simulink model "wlan_phy_rx_pmd"  (Simulink 7.8)
settings: Solver=VariableStepDiscrete  SolverMode=SingleTasking  StartTime=0.0  StopTime=rx_sim.sim_time  RelTol=1e-3
PostLoadFcn:
  | wlan_phy_rx_init
InitFcn:
  | wlan_phy_rx_init
StartFcn:
  | wlan_phy_rx_init
values from neighbouring files (symbols this model uses):
  barker_seq20 = 0.95 * circshift(barker_seq20, [0 4]) ./ max(abs(barker_seq20))    [wlan_phy_rx_init.m]
  bit_scrambler_lfsr_bytes = sum(bit_scrambler_lfsr_bytes .* repmat(2.^[0:7], 127, 1),2)    [wlan_phy_rx_init.m]
  longCorr_coef_i = [3*real(longCorr_coef)]    [wlan_phy_rx_init.m]
  longCorr_coef_q = [3*imag(longCorr_coef)]    [wlan_phy_rx_init.m]
  MAX_CP_LEN = 32    [wlan_phy_rx_init.m]
  MAX_NCBPS = NCBPS_11n_64QAM    (= 312)    [wlan_phy_rx_init.m]
  MAX_NUM_BYTES = 2^16-1    (= 65535)    [wlan_phy_rx_init.m]
  MAX_NUM_SC = 64    [wlan_phy_rx_init.m]
  MAX_NUM_SYMS = 600    [wlan_phy_rx_init.m]
  NDBPS_11a_BPSK12 = 24    [wlan_phy_rx_init.m]
  preFFT_sampBuff_numSamps = 4*MAX_NUM_SC    (= 256)    [wlan_phy_rx_init.m]
  rx_sim = struct()    [wlan_phy_rx_init.m]
  scr_ind_rev = zeros(1,128)    [wlan_phy_rx_init.m]
undefined symbols (not defined in the file or in its folder): gcbh, mcs_rom_11ag, mcs_rom_11n, wlan_rx_deinterleave_rom_gen, xlBlockLoadCallback, xlGetNormalizedPeriod, xlipgetpartsetting
libraries: xbsIndex_r4 x4943, xbsEDKLib_r4 x1
(part 18 of 28)

== system wlan_phy_rx_pmd/Sync & Antenna Sel/Pkt Det/RF B Det/Auto-Corr Pkt Det ==
  Reset OFDM : Inport
  Reset DSSS : Inport  Port=2
  rx_I : Inport  Port=3
  rx_Q : Inport  Port=4
  rx_valid : Inport  Port=5
  Auto Corr : Subsystem
  Auto Corr Pkt Det : Scope  NumInputPorts=9
      settings: TimeRange=40000  YMin=-1~-0.5~0~0~0~0~-1~0~0  YMax=1~0.5~1.5~1~0.4~1~1~1~1  SaveName=ScopeData28
  Decision : Subsystem
  Mag : Subsystem
  Register1 : xbsIndex_r4/Register  init=0  rst=off  en=off  dbl_ovrd=off  block_type=register
  Register2 : xbsIndex_r4/Register  init=0  rst=off  en=off  dbl_ovrd=off  block_type=register
  Register3 : xbsIndex_r4/Register  init=0  rst=off  en=off  dbl_ovrd=off  block_type=register
  done{1..9} (9 alike) : xbsIndex_r4/Gateway Out  inherit_from_input=off  hdl_port=off  timing_constraint=None  locs_specified=off  LOCs={}  UseAsDAC=off  DACChannel='1'  block_type=gatewayout
      settings: infoedit="Gateway out block.  Converts Xilinx fixed point inputs into ouputs of type Simulink integer, double, or fixed point.<P><P>Hardware notes:  In hardware these blocks become top level output ports or are discarded, depending on how they are configured."  xl_area=[0 0 0 0 1 0 0]  block_version=VER_STRING_GOES_HERE
  Det OFDM : Outport
  Det DSSS : Outport  Port=2
  wires:
  Reset DSSS/1 -> done9/1, Decision/2(Reset DSSS)
  Reset OFDM/1 -> done1/1, Decision/1(Reset OFDM)
  Decision/2(Det DSSS) -> Det DSSS/1, done8/1
  done9/1 -> Auto Corr Pkt Det/9  "Det Reset DSSS"
  done1/1 -> Auto Corr Pkt Det/8  "Det Reset OFDM"
  done8/1 -> Auto Corr Pkt Det/7  "Det DSSS"
  done6/1 -> Auto Corr Pkt Det/6  "Det OFDM"
  done7/1 -> Auto Corr Pkt Det/5  "Corr DSSS"
  done5/1 -> Auto Corr Pkt Det/4  "Corr OFDM"
  done3/1 -> Auto Corr Pkt Det/3  "Mag"
  done2/1 -> Auto Corr Pkt Det/2  "ADCQ"
  done4/1 -> Auto Corr Pkt Det/1  "ADCI"
  Auto Corr/2(Corr_20) -> done7/1, Decision/4(Corr_20)
  rx_Q/1 -> Register3/1
  rx_I/1 -> Register1/1
  rx_valid/1 -> Register2/1
  Register1/1 -> done4/1, Mag/2(I), Auto Corr/2(I)
  Register3/1 -> done2/1, Mag/3(Q), Auto Corr/3(Q)
  Register2/1 -> Mag/1(Valid), Auto Corr/1(Valid)
  Auto Corr/1(Corr_16) -> done5/1, Decision/3(Corr_16)
  Mag/1(Mag Sq) -> done3/1, Decision/5(Mag)
  Decision/1(Det OFDM) -> Det OFDM/1, done6/1

== system wlan_phy_rx_pmd/Sync & Antenna Sel/Pkt Det/RF B Det/RSSI Pkt Det ==
  note: The DSSS and OFDM pipelines operate independently. Both require
      | their pktDet inputs to assert at the beginning of a reception
      | and stay asserted until the pipeline is finished. But each pipeline
      | can reset the other (valid OFDM implies DSSS not valid), so
      | the pkt det state bits must be kept separate here.
  Reset OFDM : Inport
  Reset DSSS : Inport  Port=2
  Avg RSSI : Inport  Port=3
  Detection Decision : Subsystem
  Inverter : xbsIndex_r4/Inverter  en=off  latency=0  dbl_ovrd=off  block_type=inv
  Logical3 : xbsIndex_r4/Logical  logical_function=AND  inputs=2  en=off  latency=0  precision=Full  arith_type=Unsigned  n_bits=8  bin_pt=2  align_bp=on  dbl_ovrd=off  block_type=logical
      settings: xl_area=[1 0 0 1 0 0 0]  block_version=VER_STRING_GOES_HERE
  Posedge1 : Subsystem
  Register6 : xbsIndex_r4/Register  init=0  rst=off  en=off  dbl_ovrd=off  block_type=register
  S-R Latch1 : Subsystem
  S-R Latch2 : Subsystem
  PktDet OFDM : Outport
  PktDet DSSS : Outport  Port=2
  wires:
  Inverter/1 -> Register6/1
  Logical3/1 -> S-R Latch2/1(S), S-R Latch1/1(S)
  [MAC_PHY_BLOCK_PKT_DET] -> Inverter/1
  Reset DSSS/1 -> S-R Latch2/2(R)
  S-R Latch2/1(Q) -> PktDet DSSS/1
  Detection Decision/1(Pkt Det) -> Posedge1/1(D)
  Reset OFDM/1 -> S-R Latch1/2(R)
  S-R Latch1/1(Q) -> PktDet OFDM/1
  Posedge1/1(Q) -> Logical3/2
  Avg RSSI/1 -> Detection Decision/1(Avg RSSI)
  Register6/1 -> Logical3/1

== system wlan_phy_rx_pmd/Sync & Antenna Sel/Resets/Reset Gen/S-R Latch2 ==
  S : Inport
  R : Inport  Port=2
  Constant1 : xbsIndex_r4/Constant  const=1  gui_display_data_type=Fixed-point  arith_type=Boolean  n_bits=1  bin_pt=0  preci_type=Single  exp_width=8  frac_width=24  explicit_period=off  period=1  equ=P=C  opselect=C  inp2=PCIN>>17  opr=+  inp1=P  carry=CIN  dbl_ovrd=off  block_type=constant
      settings: block_version=VER_STRING_GOES_HERE
  Convert1 : xbsIndex_r4/Convert  gui_display_data_type=Boolean  arith_type=Boolean  n_bits=1  bin_pt=0  float_type=Single  exp_bits=8  fraction_bits=24  quantization=Truncate  overflow=Wrap  en=off  latency=0  dbl_ovrd=off  pipeline=off  block_type=convert
  Convert2 : xbsIndex_r4/Convert  gui_display_data_type=Boolean  arith_type=Boolean  n_bits=1  bin_pt=0  float_type=Single  exp_bits=8  fraction_bits=24  quantization=Truncate  overflow=Wrap  en=off  latency=0  dbl_ovrd=off  pipeline=off  block_type=convert
  Register2 : xbsIndex_r4/Register  init=0  rst=on  en=on  dbl_ovrd=off  block_type=register
      settings: xl_area=[1 1 0 0 0 0 0]  block_version=VER_STRING_GOES_HERE
  Q : Outport
  wires:
  Convert2/1 -> Register2/3
  Convert1/1 -> Register2/2
  Constant1/1 -> Register2/1
  R/1 -> Convert1/1
  Register2/1 -> Q/1
  S/1 -> Convert2/1

== system wlan_phy_rx_pmd/Sync & Antenna Sel/Resets/Reset Gen/negedge ==
  D : Inport
  Delay : xbsIndex_r4/Delay  rst=off  infoeditControl=Selection of Reset will increase slice count due to use of real FFs and instead of SRLs  en=off  latency=1  dbl_ovrd=off  reg_retiming=off  block_type=delay
      settings: infoedit=Hardware notes: A delay line is a chain, each link of which is an SRL16 followed by a flip-flop. If register retiming is enabled, the delay line is a chain of flip-flops.  xl_area=[1 1 0 0 0 0 0]  block_version=VER_STRING_GOES_HERE
  Inverter : xbsIndex_r4/Inverter  en=off  latency=0  dbl_ovrd=off  block_type=inv
      settings: xl_area=[0 0 0 0 0 0 0]  block_version=VER_STRING_GOES_HERE
  Logical1 : xbsIndex_r4/Logical  logical_function=AND  inputs=2  en=off  latency=0  precision=Full  arith_type=Unsigned  n_bits=8  bin_pt=2  align_bp=on  dbl_ovrd=off  block_type=logical
      settings: xl_area=[1 0 0 1 0 0 0]  block_version=VER_STRING_GOES_HERE
  Q : Outport
  wires:
  Delay/1 -> Logical1/1
  Logical1/1 -> Q/1
  D/1 -> Delay/1, Inverter/1
  Inverter/1 -> Logical1/2

== system wlan_phy_rx_pmd/Sync & Antenna Sel/Resets/Reset Gen1/S-R Latch2 ==
  S : Inport
  R : Inport  Port=2
  Constant1 : xbsIndex_r4/Constant  const=1  gui_display_data_type=Fixed-point  arith_type=Boolean  n_bits=1  bin_pt=0  preci_type=Single  exp_width=8  frac_width=24  explicit_period=off  period=1  equ=P=C  opselect=C  inp2=PCIN>>17  opr=+  inp1=P  carry=CIN  dbl_ovrd=off  block_type=constant
      settings: block_version=VER_STRING_GOES_HERE
  Convert1 : xbsIndex_r4/Convert  gui_display_data_type=Boolean  arith_type=Boolean  n_bits=1  bin_pt=0  float_type=Single  exp_bits=8  fraction_bits=24  quantization=Truncate  overflow=Wrap  en=off  latency=0  dbl_ovrd=off  pipeline=off  block_type=convert
  Convert2 : xbsIndex_r4/Convert  gui_display_data_type=Boolean  arith_type=Boolean  n_bits=1  bin_pt=0  float_type=Single  exp_bits=8  fraction_bits=24  quantization=Truncate  overflow=Wrap  en=off  latency=0  dbl_ovrd=off  pipeline=off  block_type=convert
  Register2 : xbsIndex_r4/Register  init=0  rst=on  en=on  dbl_ovrd=off  block_type=register
      settings: xl_area=[1 1 0 0 0 0 0]  block_version=VER_STRING_GOES_HERE
  Q : Outport
  wires:
  S/1 -> Convert2/1
  Register2/1 -> Q/1
  R/1 -> Convert1/1
  Constant1/1 -> Register2/1
  Convert1/1 -> Register2/2
  Convert2/1 -> Register2/3

== system wlan_phy_rx_pmd/Sync & Antenna Sel/Resets/Reset Gen1/negedge ==
  D : Inport
  Delay : xbsIndex_r4/Delay  rst=off  infoeditControl=Selection of Reset will increase slice count due to use of real FFs and instead of SRLs  en=off  latency=1  dbl_ovrd=off  reg_retiming=off  block_type=delay
      settings: infoedit=Hardware notes: A delay line is a chain, each link of which is an SRL16 followed by a flip-flop. If register retiming is enabled, the delay line is a chain of flip-flops.  xl_area=[1 1 0 0 0 0 0]  block_version=VER_STRING_GOES_HERE
  Inverter : xbsIndex_r4/Inverter  en=off  latency=0  dbl_ovrd=off  block_type=inv
      settings: xl_area=[0 0 0 0 0 0 0]  block_version=VER_STRING_GOES_HERE
  Logical1 : xbsIndex_r4/Logical  logical_function=AND  inputs=2  en=off  latency=0  precision=Full  arith_type=Unsigned  n_bits=8  bin_pt=2  align_bp=on  dbl_ovrd=off  block_type=logical
      settings: xl_area=[1 0 0 1 0 0 0]  block_version=VER_STRING_GOES_HERE
  Q : Outport
  wires:
  Inverter/1 -> Logical1/2
  D/1 -> Inverter/1, Delay/1
  Logical1/1 -> Q/1
  Delay/1 -> Logical1/1

== system wlan_phy_rx_pmd/Chan Est & EQ/Equalizer, Pilot Processing & PHY Mode Det/Phase Err Calc/Descramble/Scrambling LFSR ==
  note: Was 0!
  en : Inport
  rst : Inport  Port=2
  Assert : xbsIndex_r4/Assert  assert_type=on  type_source=Explicitly  gui_display_data_type=Boolean  arith_type=Boolean  n_bits=16  bin_pt=14  preci_type=Single  exp_width=8  frac_width=24  assert_rate=on  rate_source=Explicitly  period=1  output_port=on  block_type=assert
      settings: block_version=VER_STRING_GOES_HERE
  Assert1 : xbsIndex_r4/Assert  assert_type=on  type_source=Explicitly  gui_display_data_type=Fixed-point  arith_type=Boolean  n_bits=16  bin_pt=14  preci_type=Single  exp_width=8  frac_width=24  assert_rate=on  rate_source=Explicitly  period=1  output_port=on  block_type=assert
      settings: infoedit=Asserts a user-defined sample rate and/or type on a signal.<P><P>Hardware notes: In hardware this block costs nothing.  block_version=VER_STRING_GOES_HERE
  Convert : xbsIndex_r4/Convert  gui_display_data_type=Boolean  arith_type=Boolean  n_bits=16  bin_pt=14  float_type=Single  exp_bits=8  fraction_bits=24  quantization=Truncate  overflow=Wrap  en=off  latency=0  dbl_ovrd=off  pipeline=off  block_type=convert
  Convert1 : xbsIndex_r4/Convert  gui_display_data_type=Boolean  arith_type=Boolean  n_bits=16  bin_pt=14  float_type=Single  exp_bits=8  fraction_bits=24  quantization=Truncate  overflow=Wrap  en=off  latency=0  dbl_ovrd=off  pipeline=off  block_type=convert
  Logical : xbsIndex_r4/Logical  logical_function=XOR  inputs=2  en=off  latency=0  precision=Full  arith_type=Unsigned  n_bits=8  bin_pt=2  align_bp=on  dbl_ovrd=off  block_type=logical
      settings: xl_area=[0, 0, 0, 0, 0, 0, 0]  block_version=VER_STRING_GOES_HERE
  Register{,1..6} (7 alike) : xbsIndex_r4/Register  init=1  rst=on  en=on  dbl_ovrd=off  block_type=register
      settings: xl_area=[0, 0, 0, 0, 0, 0, 0]  block_version=VER_STRING_GOES_HERE
  Q : Outport
  wires:
  rst/1 -> Convert/1
  Register6/1 -> Assert/1
  Logical/1 -> Register/1, Q/1
  Register5/1 -> Register6/1
  Register4/1 -> Register5/1
  Register3/1 -> Register4/1, Assert1/1
  Register2/1 -> Register3/1
  Register1/1 -> Register2/1
  Register/1 -> Register1/1
  Assert/1 -> Logical/1
  Assert1/1 -> Logical/2
  en/1 -> Convert1/1
  Convert/1 -> Register/2, Register1/2, Register2/2, Register3/2, Register4/2, Register5/2, Register6/2
  Convert1/1 -> Register/3, Register1/3, Register2/3, Register3/3, Register4/3, Register5/3, Register6/3

== system wlan_phy_rx_pmd/Chan Est & EQ/Equalizer, Pilot Processing & PHY Mode Det/Phase Err Calc/Descramble/edge ==
  D : Inport
  Delay6 : xbsIndex_r4/Delay  rst=off  infoeditControl=Selection of Reset will increase slice count due to use of real FFs and instead of SRLs  en=off  latency=1  dbl_ovrd=off  reg_retiming=off  block_type=delay
  LSB : xbsIndex_r4/Slice  nbits=1  boolean_output=on  mode=Lower Bit Location + Width  bit1=0  base1=MSB of Input  bit0=0  base0=LSB of Input  dbl_ovrd=off  block_type=slice
  Logical : xbsIndex_r4/Logical  logical_function=XOR  inputs=2  en=off  latency=0  precision=Full  arith_type=Unsigned  n_bits=16  bin_pt=0  align_bp=on  dbl_ovrd=off  block_type=logical
  Q : Outport
  wires:
  LSB/1 -> Logical/1, Delay6/1
  Delay6/1 -> Logical/2
  D/1 -> LSB/1
  Logical/1 -> Q/1

== system wlan_phy_rx_pmd/DSSS Rx/Demod/Sync/SFD Search/8 Shift Reg ==
  D : Inport
  En : Inport  Port=2
  Rst : Inport  Port=3
  Concat : xbsIndex_r4/Concat  num_inputs=8  dbl_ovrd=off  block_type=concat
  Register{1..8} (8 alike) : xbsIndex_r4/Register  init=1  rst=on  en=on  dbl_ovrd=off  block_type=register
      settings: xl_area=[0, 0, 0, 0, 0, 0, 0]  block_version=VER_STRING_GOES_HERE
  Q : Outport
  8b : Outport  Port=2
  wires:
  Register1/1 -> Register2/1, Concat/2
  Register2/1 -> Register3/1, Concat/3
  Register3/1 -> Register4/1, Concat/4
  Register5/1 -> Register6/1, Concat/6
  Register6/1 -> Register7/1, Concat/7
  Register7/1 -> Register8/1, Concat/8
  Register4/1 -> Register5/1, Concat/5
  En/1 -> Register1/3, Register2/3, Register3/3, Register4/3, Register5/3, Register6/3, Register7/3, Register8/3
  Rst/1 -> Register1/2, Register7/2, Register8/2, Register6/2, Register5/2, Register4/2, Register3/2, Register2/2
  D/1 -> Register1/1, Concat/1
  Register8/1 -> Q/1
  Concat/1 -> 8b/1

== system wlan_phy_rx_pmd/DSSS Rx/Demod/Sync/SFD Search/8 Shift Reg1 ==
  D : Inport
  En : Inport  Port=2
  Rst : Inport  Port=3
  Concat : xbsIndex_r4/Concat  num_inputs=8  dbl_ovrd=off  block_type=concat
  Register{1..8} (8 alike) : xbsIndex_r4/Register  init=1  rst=on  en=on  dbl_ovrd=off  block_type=register
      settings: xl_area=[0, 0, 0, 0, 0, 0, 0]  block_version=VER_STRING_GOES_HERE
  Q : Outport
  8b : Outport  Port=2
  wires:
  Concat/1 -> 8b/1
  Register8/1 -> Q/1
  D/1 -> Concat/1, Register1/1
  Rst/1 -> Register2/2, Register3/2, Register4/2, Register5/2, Register6/2, Register8/2, Register7/2, Register1/2
  En/1 -> Register8/3, Register7/3, Register6/3, Register5/3, Register4/3, Register3/3, Register2/3, Register1/3
  Register4/1 -> Concat/5, Register5/1
  Register7/1 -> Concat/8, Register8/1
  Register6/1 -> Concat/7, Register7/1
  Register5/1 -> Concat/6, Register6/1
  Register3/1 -> Concat/4, Register4/1
  Register2/1 -> Concat/3, Register3/1
  Register1/1 -> Concat/2, Register2/1

== system wlan_phy_rx_pmd/DSSS Rx/Demod/Sync/SFD Search/Posedge ==
  D : Inport
  Delay : xbsIndex_r4/Delay  rst=off  infoeditControl=Selection of Reset will increase slice count due to use of real FFs and instead of SRLs  en=off  latency=1  dbl_ovrd=off  reg_retiming=off  block_type=delay
      settings: infoedit=Hardware notes: A delay line is a chain, each link of which is an SRL16 followed by a flip-flop. If register retiming is enabled, the delay line is a chain of flip-flops.  xl_area=[1 1 0 0 0 0 0]  block_version=VER_STRING_GOES_HERE
  Inverter : xbsIndex_r4/Inverter  en=off  latency=0  dbl_ovrd=off  block_type=inv
      settings: xl_area=[0 0 0 0 0 0 0]  block_version=VER_STRING_GOES_HERE
  Logical1 : xbsIndex_r4/Logical  logical_function=AND  inputs=2  en=off  latency=0  precision=Full  arith_type=Unsigned  n_bits=8  bin_pt=2  align_bp=on  dbl_ovrd=off  block_type=logical
      settings: xl_area=[1 0 0 1 0 0 0]  block_version=VER_STRING_GOES_HERE
  Q : Outport
  wires:
  D/1 -> Logical1/2, Delay/1
  Logical1/1 -> Q/1
  Inverter/1 -> Logical1/1
  Delay/1 -> Inverter/1

== system wlan_phy_rx_pmd/Detect & Decode/De-Interleave/Read Addr Gen/Ctrl/Bit Index Calc ==
  note: Accumulator increments by 1 or 2 bits per cycle, depending
      | on whether current LLR pair had a punctured value.
      | Outputs are used as memory addresses in de-interleaver
      | mapping ROMs. PHY Mode (11ag vs 11n) is MSB,
      | modulation index is next, then the actual bit indexes.
  Mod : Inport
  SIGNAL Sym : Inport  Port=2
  Inc : Inport  Port=3
  Rst : Inport  Port=4
  En : Inport  Port=5
  Accumulator : xbsIndex_r4/Accumulator  operation=Add  n_bits=ceil(log2(MAX_NCBPS))  overflow=Wrap  scale=1  rst=on  infoeditControl=reset for floating point data type must be asserted for a minimum of 2 cycles  hasbypass=off  en=on  latency=0  dbl_ovrd=off  msb_inp=100  msb=100  lsb=-100  use_behavioral_HDL=on  implementation=Fabric  block_type=accum
  AddSub : xbsIndex_r4/AddSub  mode=Addition  use_carryin=off  use_carryout=off  en=off  latency=0  precision=User Defined  arith_type=Unsigned  n_bits=ceil(log2(MAX_NCBPS))  bin_pt=0  quantization=Truncate  overflow=Wrap  dbl_ovrd=off  use_behavioral_HDL=off  hw_selection=Fabric  pipelined=off  block_type=addsub
  Concat : xbsIndex_r4/Concat  num_inputs=3  dbl_ovrd=off  block_type=concat
  Concat1 : xbsIndex_r4/Concat  num_inputs=3  dbl_ovrd=off  block_type=concat
  Constant2 : xbsIndex_r4/Constant  const=1  gui_display_data_type=Fixed-point  arith_type=Unsigned  n_bits=1  bin_pt=0  preci_type=Single  exp_width=8  frac_width=24  explicit_period=off  period=1  equ=P=C  opselect=C  inp2=PCIN>>17  opr=+  inp1=P  carry=CIN  dbl_ovrd=off  block_type=constant
  Inverter : xbsIndex_r4/Inverter  en=off  latency=0  dbl_ovrd=off  block_type=inv
  Logical : xbsIndex_r4/Logical  logical_function=AND  inputs=2  en=off  latency=0  precision=Full  arith_type=Unsigned  n_bits=16  bin_pt=0  align_bp=on  dbl_ovrd=off  block_type=logical
  Ind A : Outport
  Ind B : Outport  Port=2
  wires:
  Accumulator/1 -> AddSub/1, Concat/3
  Concat/1 -> Ind A/1
  Concat1/1 -> Ind B/1
  AddSub/1 -> Concat1/3
  Constant2/1 -> AddSub/2
  Inverter/1 -> Logical/1
  [RX_PHY_MODE_11N] -> Logical/2
  Logical/1 -> Concat/1, Concat1/1
  SIGNAL Sym/1 -> Inverter/1
  Mod/1 -> Concat/2, Concat1/2
  Rst/1 -> Accumulator/2
  En/1 -> Accumulator/3
  Inc/1 -> Accumulator/1

== system wlan_phy_rx_pmd/Detect & Decode/De-Interleave/Read Addr Gen/Ctrl/Data Bits Count ==
  note: This subsystem counts how many LLR pairs are input to
      | the decoder. Each LLR pair encodes 2 coded bits (1 data bit). It would be possible
      | to replace this block with a more efficient one based on the control
      | blocks one level up, but this design will enable easier addition of
      | additional rates in the future.
  note: IMPORTANT: the N_DBPS values used here assume 1 spatial stream.
      | These values represent the number of data bits in a single OFDM symbol,
      | *not* the number of bits in each OFDM symbol interval. When this model
      | is extended to 2 spatial streams this block will require modifications
      | to divide N_DBPS by 2 when N_SS = 2!
  En : Inport
  SIGNAL sym : Inport  Port=2
  AddSub : xbsIndex_r4/AddSub  mode=Subtraction  use_carryin=off  use_carryout=off  en=off  latency=1  precision=Full  arith_type=Unsigned  n_bits=16  bin_pt=14  quantization=Truncate  overflow=Wrap  dbl_ovrd=off  use_behavioral_HDL=off  hw_selection=Fabric  pipelined=off  block_type=addsub
  Constant17 : xbsIndex_r4/Constant  const=1  gui_display_data_type=Fixed-point  arith_type=Unsigned  n_bits=1  bin_pt=0  preci_type=Single  exp_width=8  frac_width=24  explicit_period=on  period=1  equ=P=C  opselect=C  inp2=PCIN>>17  opr=+  inp1=P  carry=CIN  dbl_ovrd=off  block_type=constant
  Constant19 : xbsIndex_r4/Constant  const=NDBPS_11a_BPSK12-1  gui_display_data_type=Fixed-point  arith_type=Unsigned  n_bits=ceil(log2(MAX_NCBPS))  bin_pt=0  preci_type=Single  exp_width=8  frac_width=24  explicit_period=off  period=1  equ=P=C  opselect=C  inp2=PCIN>>17  opr=+  inp1=P  carry=CIN  dbl_ovrd=off  block_type=constant
  Counter : xbsIndex_r4/Counter  cnt_type=Free Running  cnt_to=Inf  operation=Up  start_count=0  cnt_by_val=1  arith_type=Unsigned  n_bits=ceil(log2(MAX_NCBPS))  bin_pt=0  load_pin=off  rst=on  en=on  explicit_period=off  period=1  dbl_ovrd=off  use_behavioral_HDL=off  implementation=Fabric  block_type=counter
  Mux4 : xbsIndex_r4/Mux  inputs=2  en=off  latency=0  precision=Full  arith_type=Unsigned  n_bits=16  bin_pt=14  quantization=Truncate  overflow=Wrap  dbl_ovrd=off  block_type=mux
  Relational1 : xbsIndex_r4/Relational  mode=a>=b  en=off  latency=0  dbl_ovrd=off  block_type=relational
  Done : Outport
  wires:
  Relational1/1 -> Done/1, Counter/1
  Counter/1 -> Relational1/1
  En/1 -> Counter/2
  SIGNAL sym/1 -> Mux4/1
  Constant19/1 -> Mux4/3
  [OFDM_RX_DATA_N_DBPS_2] -> AddSub/1
  Constant17/1 -> AddSub/2
  AddSub/1 -> Mux4/2
  Mux4/1 -> Relational1/2

== system wlan_phy_rx_pmd/Detect & Decode/De-Interleave/Read Addr Gen/Ctrl/Rate 1/2 ==
  Rst : Inport
  En : Inport  Port=2
  Constant2 : xbsIndex_r4/Constant  const=0  gui_display_data_type=Boolean  arith_type=Boolean  n_bits=2  bin_pt=0  preci_type=Single  exp_width=8  frac_width=24  explicit_period=on  period=1  equ=P=C  opselect=C  inp2=PCIN>>17  opr=+  inp1=P  carry=CIN  dbl_ovrd=off  block_type=constant
  Constant3 : xbsIndex_r4/Constant  const=2  gui_display_data_type=Fixed-point  arith_type=Unsigned  n_bits=2  bin_pt=0  preci_type=Single  exp_width=8  frac_width=24  explicit_period=off  period=1  equ=P=C  opselect=C  inp2=PCIN>>17  opr=+  inp1=P  carry=CIN  dbl_ovrd=off  block_type=constant
  Ind Inc : Outport
  Skip A : Outport  Port=2
  Skip B : Outport  Port=3
  wires:
  Constant2/1 -> Skip A/1, Skip B/1
  Constant3/1 -> Ind Inc/1

== system wlan_phy_rx_pmd/Detect & Decode/De-Interleave/Read Addr Gen/Ctrl/Rate 2/3 ==
  Rst : Inport
  En : Inport  Port=2
  Constant1 : xbsIndex_r4/Constant  const=1  gui_display_data_type=Fixed-point  arith_type=Unsigned  n_bits=2  bin_pt=0  preci_type=Single  exp_width=8  frac_width=24  explicit_period=off  period=1  equ=P=C  opselect=C  inp2=PCIN>>17  opr=+  inp1=P  carry=CIN  dbl_ovrd=off  block_type=constant
  Constant2 : xbsIndex_r4/Constant  const=0  gui_display_data_type=Boolean  arith_type=Boolean  n_bits=2  bin_pt=0  preci_type=Single  exp_width=8  frac_width=24  explicit_period=on  period=1  equ=P=C  opselect=C  inp2=PCIN>>17  opr=+  inp1=P  carry=CIN  dbl_ovrd=off  block_type=constant
  Constant3 : xbsIndex_r4/Constant  const=2  gui_display_data_type=Fixed-point  arith_type=Unsigned  n_bits=2  bin_pt=0  preci_type=Single  exp_width=8  frac_width=24  explicit_period=off  period=1  equ=P=C  opselect=C  inp2=PCIN>>17  opr=+  inp1=P  carry=CIN  dbl_ovrd=off  block_type=constant
  Constant4 : xbsIndex_r4/Constant  const=1  gui_display_data_type=Fixed-point  arith_type=Unsigned  n_bits=2  bin_pt=0  preci_type=Single  exp_width=8  frac_width=24  explicit_period=off  period=1  equ=P=C  opselect=C  inp2=PCIN>>17  opr=+  inp1=P  carry=CIN  dbl_ovrd=off  block_type=constant
  Mux3 : xbsIndex_r4/Mux  inputs=2  en=off  latency=0  precision=Full  arith_type=Unsigned  n_bits=16  bin_pt=14  quantization=Truncate  overflow=Wrap  dbl_ovrd=off  block_type=mux
  Relational : xbsIndex_r4/Relational  mode=a=b  en=off  latency=0  dbl_ovrd=off  block_type=relational
  "[0:1]" : xbsIndex_r4/Counter  cnt_type=Free Running  cnt_to=5  operation=Up  start_count=0  cnt_by_val=1  arith_type=Unsigned  n_bits=1  bin_pt=0  load_pin=off  rst=on  en=on  explicit_period=off  period=1  dbl_ovrd=off  use_behavioral_HDL=off  implementation=Fabric  block_type=counter
  Ind Inc : Outport
  Skip A : Outport  Port=2
  Skip B : Outport  Port=3
  wires:
  "[0:1]"/1 -> Relational/1
  Constant1/1 -> Relational/2
  Relational/1 -> Skip B/1, Mux3/1
  Rst/1 -> "[0:1]"/1
  En/1 -> "[0:1]"/2
  Constant2/1 -> Skip A/1
  Mux3/1 -> Ind Inc/1
  Constant3/1 -> Mux3/2
  Constant4/1 -> Mux3/3

== system wlan_phy_rx_pmd/Detect & Decode/De-Interleave/Read Addr Gen/Ctrl/Rate 3/4 ==
  Rst : Inport
  En : Inport  Port=2
  Concat : xbsIndex_r4/Concat  num_inputs=2  dbl_ovrd=off  block_type=concat
  Constant1 : xbsIndex_r4/Constant  const=2  gui_display_data_type=Fixed-point  arith_type=Unsigned  n_bits=2  bin_pt=0  preci_type=Single  exp_width=8  frac_width=24  explicit_period=off  period=1  equ=P=C  opselect=C  inp2=PCIN>>17  opr=+  inp1=P  carry=CIN  dbl_ovrd=off  block_type=constant
  Constant2 : xbsIndex_r4/Constant  const=1  gui_display_data_type=Fixed-point  arith_type=Unsigned  n_bits=1  bin_pt=0  preci_type=Single  exp_width=8  frac_width=24  explicit_period=off  period=1  equ=P=C  opselect=C  inp2=PCIN>>17  opr=+  inp1=P  carry=CIN  dbl_ovrd=off  block_type=constant
  Constant3 : xbsIndex_r4/Constant  const=2  gui_display_data_type=Fixed-point  arith_type=Unsigned  n_bits=2  bin_pt=0  preci_type=Single  exp_width=8  frac_width=24  explicit_period=off  period=1  equ=P=C  opselect=C  inp2=PCIN>>17  opr=+  inp1=P  carry=CIN  dbl_ovrd=off  block_type=constant
  Constant4 : xbsIndex_r4/Constant  const=2  gui_display_data_type=Fixed-point  arith_type=Unsigned  n_bits=2  bin_pt=0  preci_type=Single  exp_width=8  frac_width=24  explicit_period=off  period=1  equ=P=C  opselect=C  inp2=PCIN>>17  opr=+  inp1=P  carry=CIN  dbl_ovrd=off  block_type=constant
  Constant7 : xbsIndex_r4/Constant  const=0  gui_display_data_type=Fixed-point  arith_type=Unsigned  n_bits=2  bin_pt=0  preci_type=Single  exp_width=8  frac_width=24  explicit_period=off  period=1  equ=P=C  opselect=C  inp2=PCIN>>17  opr=+  inp1=P  carry=CIN  dbl_ovrd=off  block_type=constant
  Mux3 : xbsIndex_r4/Mux  inputs=3  en=off  latency=0  precision=Full  arith_type=Unsigned  n_bits=16  bin_pt=14  quantization=Truncate  overflow=Wrap  dbl_ovrd=off  block_type=mux
  Relational : xbsIndex_r4/Relational  mode=a=b  en=off  latency=0  dbl_ovrd=off  block_type=relational
  Relational2 : xbsIndex_r4/Relational  mode=a=b  en=off  latency=0  dbl_ovrd=off  block_type=relational
  "[0:2]" : xbsIndex_r4/Counter  cnt_type=Count Limited  cnt_to=2  operation=Up  start_count=0  cnt_by_val=1  arith_type=Unsigned  n_bits=2  bin_pt=0  load_pin=off  rst=on  en=on  explicit_period=off  period=1  dbl_ovrd=off  use_behavioral_HDL=off  implementation=Fabric  block_type=counter
  Ind Inc : Outport
  Skip A : Outport  Port=2
  Skip B : Outport  Port=3
  wires:
  En/1 -> "[0:2]"/2
  Rst/1 -> "[0:2]"/1
  Relational2/1 -> Skip B/1, Concat/1
  Relational/1 -> Skip A/1, Concat/2
  Constant2/1 -> Relational2/2
  Constant1/1 -> Relational/2
  "[0:2]"/1 -> Relational2/1, Relational/1
  Mux3/1 -> Ind Inc/1
  Constant3/1 -> Mux3/2
  Constant4/1 -> Mux3/3
  Concat/1 -> Mux3/1
  Constant7/1 -> Mux3/4

== system wlan_phy_rx_pmd/Detect & Decode/De-Interleave/Read Addr Gen/Ctrl/Rate 5/6 ==
  Rst : Inport
  En : Inport  Port=2
  Concat : xbsIndex_r4/Concat  num_inputs=2  dbl_ovrd=off  block_type=concat
  Constant1 : xbsIndex_r4/Constant  const=1  gui_display_data_type=Fixed-point  arith_type=Unsigned  n_bits=2  bin_pt=0  preci_type=Single  exp_width=8  frac_width=24  explicit_period=off  period=1  equ=P=C  opselect=C  inp2=PCIN>>17  opr=+  inp1=P  carry=CIN  dbl_ovrd=off  block_type=constant
  Constant2 : xbsIndex_r4/Constant  const=2  gui_display_data_type=Fixed-point  arith_type=Unsigned  n_bits=2  bin_pt=0  preci_type=Single  exp_width=8  frac_width=24  explicit_period=off  period=1  equ=P=C  opselect=C  inp2=PCIN>>17  opr=+  inp1=P  carry=CIN  dbl_ovrd=off  block_type=constant
  Constant3 : xbsIndex_r4/Constant  const=2  gui_display_data_type=Fixed-point  arith_type=Unsigned  n_bits=2  bin_pt=0  preci_type=Single  exp_width=8  frac_width=24  explicit_period=off  period=1  equ=P=C  opselect=C  inp2=PCIN>>17  opr=+  inp1=P  carry=CIN  dbl_ovrd=off  block_type=constant
  Constant4 : xbsIndex_r4/Constant  const=2  gui_display_data_type=Fixed-point  arith_type=Unsigned  n_bits=2  bin_pt=0  preci_type=Single  exp_width=8  frac_width=24  explicit_period=off  period=1  equ=P=C  opselect=C  inp2=PCIN>>17  opr=+  inp1=P  carry=CIN  dbl_ovrd=off  block_type=constant
  Constant5 : xbsIndex_r4/Constant  const=3  gui_display_data_type=Fixed-point  arith_type=Unsigned  n_bits=2  bin_pt=0  preci_type=Single  exp_width=8  frac_width=24  explicit_period=off  period=1  equ=P=C  opselect=C  inp2=PCIN>>17  opr=+  inp1=P  carry=CIN  dbl_ovrd=off  block_type=constant
  Constant6 : xbsIndex_r4/Constant  const=4  gui_display_data_type=Fixed-point  arith_type=Unsigned  n_bits=3  bin_pt=0  preci_type=Single  exp_width=8  frac_width=24  explicit_period=off  period=1  equ=P=C  opselect=C  inp2=PCIN>>17  opr=+  inp1=P  carry=CIN  dbl_ovrd=off  block_type=constant
  Constant7 : xbsIndex_r4/Constant  const=0  gui_display_data_type=Fixed-point  arith_type=Unsigned  n_bits=2  bin_pt=0  preci_type=Single  exp_width=8  frac_width=24  explicit_period=off  period=1  equ=P=C  opselect=C  inp2=PCIN>>17  opr=+  inp1=P  carry=CIN  dbl_ovrd=off  block_type=constant
  Logical : xbsIndex_r4/Logical  logical_function=OR  inputs=2  en=off  latency=0  precision=Full  arith_type=Unsigned  n_bits=16  bin_pt=0  align_bp=on  dbl_ovrd=off  block_type=logical
  Logical1 : xbsIndex_r4/Logical  logical_function=OR  inputs=2  en=off  latency=0  precision=Full  arith_type=Unsigned  n_bits=16  bin_pt=0  align_bp=on  dbl_ovrd=off  block_type=logical
  Mux3 : xbsIndex_r4/Mux  inputs=3  en=off  latency=0  precision=Full  arith_type=Unsigned  n_bits=16  bin_pt=14  quantization=Truncate  overflow=Wrap  dbl_ovrd=off  block_type=mux
  Relational{,1..3} (4 alike) : xbsIndex_r4/Relational  mode=a=b  en=off  latency=0  dbl_ovrd=off  block_type=relational
  "[0:4]" : xbsIndex_r4/Counter  cnt_type=Count Limited  cnt_to=4  operation=Up  start_count=0  cnt_by_val=1  arith_type=Unsigned  n_bits=3  bin_pt=0  load_pin=off  rst=on  en=on  explicit_period=off  period=1  dbl_ovrd=off  use_behavioral_HDL=off  implementation=Fabric  block_type=counter
  Ind Inc : Outport
  Skip A : Outport  Port=2
  Skip B : Outport  Port=3
  wires:
  Constant7/1 -> Mux3/4
  Concat/1 -> Mux3/1
  Constant4/1 -> Mux3/3
  Constant3/1 -> Mux3/2
  Mux3/1 -> Ind Inc/1
  "[0:4]"/1 -> Relational/1, Relational2/1, Relational1/1, Relational3/1
  Constant2/1 -> Relational/2
  Constant6/1 -> Relational2/2
  Rst/1 -> "[0:4]"/1
  En/1 -> "[0:4]"/2
  Constant1/1 -> Relational1/2
  Constant5/1 -> Relational3/2
  Relational/1 -> Logical/1
  Relational2/1 -> Logical/2
  Logical/1 -> Skip A/1, Concat/2
  Relational1/1 -> Logical1/1
  Relational3/1 -> Logical1/2
  Logical1/1 -> Skip B/1, Concat/1

== system wlan_phy_rx_pmd/Detect & Decode/Decode/Control/Early Trace Ctrl/S-R Latch1 ==
  S : Inport
  R : Inport  Port=2
  Constant1 : xbsIndex_r4/Constant  const=1  gui_display_data_type=Fixed-point  arith_type=Unsigned  n_bits=1  bin_pt=0  preci_type=Single  exp_width=8  frac_width=24  explicit_period=off  period=1  equ=P=C  opselect=C  inp2=PCIN>>17  opr=+  inp1=P  carry=CIN  dbl_ovrd=off  block_type=constant
      settings: block_version=VER_STRING_GOES_HERE
  Convert1 : xbsIndex_r4/Convert  gui_display_data_type=Boolean  arith_type=Boolean  n_bits=1  bin_pt=0  float_type=Single  exp_bits=8  fraction_bits=24  quantization=Truncate  overflow=Wrap  en=off  latency=0  dbl_ovrd=off  pipeline=off  block_type=convert
  Convert2 : xbsIndex_r4/Convert  gui_display_data_type=Boolean  arith_type=Boolean  n_bits=1  bin_pt=0  float_type=Single  exp_bits=8  fraction_bits=24  quantization=Truncate  overflow=Wrap  en=off  latency=0  dbl_ovrd=off  pipeline=off  block_type=convert
  Register2 : xbsIndex_r4/Register  init=0  rst=on  en=on  dbl_ovrd=off  block_type=register
      settings: xl_area=[1 1 0 0 0 0 0]  block_version=VER_STRING_GOES_HERE
  Q : Outport
  wires:
  Convert2/1 -> Register2/3
  Convert1/1 -> Register2/2
  Constant1/1 -> Register2/1
  R/1 -> Convert1/1
  Register2/1 -> Q/1
  S/1 -> Convert2/1

== system wlan_phy_rx_pmd/Detect & Decode/Decode/Control/Early Trace Ctrl/S-R Latch3 ==
  S : Inport
  R : Inport  Port=2
  Constant1 : xbsIndex_r4/Constant  const=1  gui_display_data_type=Fixed-point  arith_type=Unsigned  n_bits=1  bin_pt=0  preci_type=Single  exp_width=8  frac_width=24  explicit_period=off  period=1  equ=P=C  opselect=C  inp2=PCIN>>17  opr=+  inp1=P  carry=CIN  dbl_ovrd=off  block_type=constant
      settings: block_version=VER_STRING_GOES_HERE
  Convert1 : xbsIndex_r4/Convert  gui_display_data_type=Boolean  arith_type=Boolean  n_bits=1  bin_pt=0  float_type=Single  exp_bits=8  fraction_bits=24  quantization=Truncate  overflow=Wrap  en=off  latency=0  dbl_ovrd=off  pipeline=off  block_type=convert
  Convert2 : xbsIndex_r4/Convert  gui_display_data_type=Boolean  arith_type=Boolean  n_bits=1  bin_pt=0  float_type=Single  exp_bits=8  fraction_bits=24  quantization=Truncate  overflow=Wrap  en=off  latency=0  dbl_ovrd=off  pipeline=off  block_type=convert
  Register2 : xbsIndex_r4/Register  init=0  rst=on  en=on  dbl_ovrd=off  block_type=register
      settings: xl_area=[1 1 0 0 0 0 0]  block_version=VER_STRING_GOES_HERE
  Q : Outport
  wires:
  S/1 -> Convert2/1
  Register2/1 -> Q/1
  R/1 -> Convert1/1
  Constant1/1 -> Register2/1
  Convert1/1 -> Register2/2
  Convert2/1 -> Register2/3

== system wlan_phy_rx_pmd/Detect & Decode/Decode/Control/Early Trace Ctrl/posedge ==
  D : Inport
  Delay : xbsIndex_r4/Delay  rst=off  infoeditControl=Selection of Reset will increase slice count due to use of real FFs and instead of SRLs  en=off  latency=1  dbl_ovrd=off  reg_retiming=off  block_type=delay
      settings: infoedit=Hardware notes: A delay line is a chain, each link of which is an SRL16 followed by a flip-flop. If register retiming is enabled, the delay line is a chain of flip-flops.  xl_area=[1 1 0 0 0 0 0]  block_version=VER_STRING_GOES_HERE
  Inverter : xbsIndex_r4/Inverter  en=off  latency=0  dbl_ovrd=off  block_type=inv
      settings: xl_area=[0 0 0 0 0 0 0]  block_version=VER_STRING_GOES_HERE
  Logical1 : xbsIndex_r4/Logical  logical_function=AND  inputs=2  en=off  latency=0  precision=Full  arith_type=Unsigned  n_bits=8  bin_pt=2  align_bp=on  dbl_ovrd=off  block_type=logical
      settings: xl_area=[1 0 0 1 0 0 0]  block_version=VER_STRING_GOES_HERE
  Q : Outport
  wires:
  Inverter/1 -> Logical1/2
  Logical1/1 -> Q/1
  D/1 -> Delay/1, Logical1/1
  Delay/1 -> Inverter/1

== system wlan_phy_rx_pmd/Detect & Decode/Decode/Control/Early Trace Ctrl/posedge1 ==
  D : Inport
  Delay : xbsIndex_r4/Delay  rst=off  infoeditControl=Selection of Reset will increase slice count due to use of real FFs and instead of SRLs  en=off  latency=1  dbl_ovrd=off  reg_retiming=off  block_type=delay
      settings: infoedit=Hardware notes: A delay line is a chain, each link of which is an SRL16 followed by a flip-flop. If register retiming is enabled, the delay line is a chain of flip-flops.  xl_area=[1 1 0 0 0 0 0]  block_version=VER_STRING_GOES_HERE
  Inverter : xbsIndex_r4/Inverter  en=off  latency=0  dbl_ovrd=off  block_type=inv
      settings: xl_area=[0 0 0 0 0 0 0]  block_version=VER_STRING_GOES_HERE
  Logical1 : xbsIndex_r4/Logical  logical_function=AND  inputs=2  en=off  latency=0  precision=Full  arith_type=Unsigned  n_bits=8  bin_pt=2  align_bp=on  dbl_ovrd=off  block_type=logical
      settings: xl_area=[1 0 0 1 0 0 0]  block_version=VER_STRING_GOES_HERE
  Q : Outport
  wires:
  Delay/1 -> Inverter/1
  D/1 -> Logical1/1, Delay/1
  Logical1/1 -> Q/1
  Inverter/1 -> Logical1/2

== system wlan_phy_rx_pmd/Detect & Decode/Decode/SIGNAL & HT-SIG Decode/HT-SIG Decode/CRC8 Calc ==
  note: HT-SIG is 6 bytes (48 bits) long.
      | Bits 34:41 are an inverted 8-bit checkusm calculated over bits 0:33.
      | This subsystem is a parallel version of the serial CRC calculation shown in IEEE 802.11-2012 Figure 20-8.
      | This block consumes the 6 bytes of the HT-SIG. A valid checksum will result in the crc_accum register
      | being 0 after the last input byte.
      | Bytes 7 and 8 are pre-processed:
      | B7: the 6 bits of CRC in byte 7 are inverted, reversing the inversion at the Tx CRC calc
      | B8: the 2 bits of CRC in byte 8 are inverted. The remaining bits are set to zero. If the CRC of the first 42
      |   bits (data+crc) is valid crc_accum will be 0 and these 6 zeros will not change the crc value.
  byte_ind : Inport
  data_valid : Inport  Port=2
  data_byte : Inport  Port=3
  reset : Inport  Port=4
  2LSB2 : xbsIndex_r4/Slice  nbits=2  boolean_output=off  mode=Lower Bit Location + Width  bit1=0  base1=MSB of Input  bit0=0  base0=LSB of Input  dbl_ovrd=off  block_type=slice
  2LSB3 : xbsIndex_r4/Slice  nbits=2  boolean_output=off  mode=Lower Bit Location + Width  bit1=0  base1=MSB of Input  bit0=0  base0=LSB of Input  dbl_ovrd=off  block_type=slice
  6MSB : xbsIndex_r4/Slice  nbits=6  boolean_output=off  mode=Upper Bit Location + Width  bit1=0  base1=MSB of Input  bit0=0  base0=LSB of Input  dbl_ovrd=off  block_type=slice
  Assert1 : xbsIndex_r4/Assert  assert_type=off  type_source=Explicitly  gui_display_data_type=Fixed-point  arith_type="Signed  (2's comp)"  n_bits=16  bin_pt=14  preci_type=Single  exp_width=8  frac_width=24  assert_rate=on  rate_source=Explicitly  period=1  output_port=on  block_type=assert
      settings: block_version=VER_STRING_GOES_HERE
  CRC8 Lookup : xbsIndex_r4/ROM  depth=256  initVector=CRC_Table8  distributed_mem=Distributed memory  rst=off  init_reg=0  en=off  latency=0  gui_display_data_type=Fixed-point  arith_type=Unsigned  n_bits=8  bin_pt=0  preci_type=Single  exp_width=8  frac_width=24  dbl_ovrd=off  optimize=Area  use_rpm=off  block_type=sprom
      settings: xl_area=[0, 0, 0, 0, 0, 0, 0]  block_version=VER_STRING_GOES_HERE
  Concat1 : xbsIndex_r4/Concat  num_inputs=2  dbl_ovrd=off  block_type=concat
  Concat2 : xbsIndex_r4/Concat  num_inputs=2  dbl_ovrd=off  block_type=concat
  Concat4 : xbsIndex_r4/Concat  num_inputs=2  dbl_ovrd=off  block_type=concat
  Constant : xbsIndex_r4/Constant  const=0  gui_display_data_type=Fixed-point  arith_type=Unsigned  n_bits=8  bin_pt=0  preci_type=Single  exp_width=8  frac_width=24  explicit_period=off  period=1  equ=P=C  opselect=C  inp2=PCIN>>17  opr=+  inp1=P  carry=CIN  dbl_ovrd=off  block_type=constant
  Constant1 : xbsIndex_r4/Constant  const=0  gui_display_data_type=Fixed-point  arith_type=Unsigned  n_bits=6  bin_pt=0  preci_type=Single  exp_width=8  frac_width=24  explicit_period=off  period=1  equ=P=C  opselect=C  inp2=PCIN>>17  opr=+  inp1=P  carry=CIN  dbl_ovrd=off  block_type=constant
  Constant2 : xbsIndex_r4/Constant  const=8  gui_display_data_type=Fixed-point  arith_type=Unsigned  n_bits=4  bin_pt=0  preci_type=Single  exp_width=8  frac_width=24  explicit_period=off  period=1  equ=P=C  opselect=C  inp2=PCIN>>17  opr=+  inp1=P  carry=CIN  dbl_ovrd=off  block_type=constant
  Constant3 : xbsIndex_r4/Constant  const=3  gui_display_data_type=Fixed-point  arith_type=Unsigned  n_bits=4  bin_pt=0  preci_type=Single  exp_width=8  frac_width=24  explicit_period=off  period=1  equ=P=C  opselect=C  inp2=PCIN>>17  opr=+  inp1=P  carry=CIN  dbl_ovrd=off  block_type=constant
  Constant4 : xbsIndex_r4/Constant  const=8  gui_display_data_type=Fixed-point  arith_type=Unsigned  n_bits=4  bin_pt=0  preci_type=Single  exp_width=8  frac_width=24  explicit_period=off  period=1  equ=P=C  opselect=C  inp2=PCIN>>17  opr=+  inp1=P  carry=CIN  dbl_ovrd=off  block_type=constant
  Constant5 : xbsIndex_r4/Constant  const=7  gui_display_data_type=Fixed-point  arith_type=Unsigned  n_bits=3  bin_pt=0  preci_type=Single  exp_width=8  frac_width=24  explicit_period=off  period=1  equ=P=C  opselect=C  inp2=PCIN>>17  opr=+  inp1=P  carry=CIN  dbl_ovrd=off  block_type=constant
  Delay : xbsIndex_r4/Delay  rst=off  infoeditControl=Selection of Reset will increase slice count due to use of real FFs and instead of SRLs  en=off  latency=1  dbl_ovrd=off  reg_retiming=off  block_type=delay
  Endian Swap : Subsystem
  Gateway Out{1..4,10} (5 alike) : xbsIndex_r4/Gateway Out  inherit_from_input=off  hdl_port=off  timing_constraint=None  locs_specified=off  LOCs={}  UseAsDAC=off  DACChannel='1'  block_type=gatewayout
  HT-SIG CRC : Scope  NumInputPorts=8
      settings: TimeRange=15000  YMin=0~0~0~0~0~-1~-1~-1  YMax=1~1~250~1~300~1~1~1  SaveName=ScopeData15
  Inverter : xbsIndex_r4/Inverter  en=off  latency=0  dbl_ovrd=off  block_type=inv
  Inverter1 : xbsIndex_r4/Inverter  en=off  latency=0  dbl_ovrd=off  block_type=inv
  Logical1 : xbsIndex_r4/Logical  logical_function=AND  inputs=3  en=off  latency=0  precision=Full  arith_type=Unsigned  n_bits=16  bin_pt=0  align_bp=on  dbl_ovrd=off  block_type=logical
  Logical3 : xbsIndex_r4/Logical  logical_function=XOR  inputs=2  en=off  latency=0  precision=Full  arith_type=Unsigned  n_bits=8  bin_pt=2  align_bp=on  dbl_ovrd=off  block_type=logical
      settings: xl_area=[0, 0, 0, 0, 0, 0, 0]  block_version=VER_STRING_GOES_HERE
  Mux : xbsIndex_r4/Mux  inputs=3  en=off  latency=0  precision=Full  arith_type=Unsigned  n_bits=16  bin_pt=14  quantization=Truncate  overflow=Wrap  dbl_ovrd=off  block_type=mux
  Relational1 : xbsIndex_r4/Relational  mode=a=b  en=off  latency=0  dbl_ovrd=off  block_type=relational
  Relational2 : xbsIndex_r4/Relational  mode=a=b  en=off  latency=0  dbl_ovrd=off  block_type=relational
  Relational3 : xbsIndex_r4/Relational  mode=a>=b  en=off  latency=0  dbl_ovrd=off  block_type=relational
  Relational4 : xbsIndex_r4/Relational  mode=a<=b  en=off  latency=0  dbl_ovrd=off  block_type=relational
  Relational5 : xbsIndex_r4/Relational  mode=a=b  en=off  latency=0  dbl_ovrd=off  block_type=relational
  byte : xbsIndex_r4/Register  init=0  rst=on  en=on  dbl_ovrd=off  block_type=register
      settings: xl_area=[0, 0, 0, 0, 0, 0, 0]  block_version=VER_STRING_GOES_HERE
  crc_accum : xbsIndex_r4/Register  init=hex2dec('FF') % ('27')  rst=on  en=on  dbl_ovrd=off  block_type=register
      settings: xl_area=[0, 0, 0, 0, 0, 0, 0]  block_version=VER_STRING_GOES_HERE
  CRC Good : Outport
  wires:
  Constant1/1 -> Concat2/1
  Inverter1/1 -> Concat2/2
  2LSB3/1 -> Inverter1/1
  2LSB2/1 -> Concat4/2
  Inverter/1 -> Concat4/1
  6MSB/1 -> Inverter/1
  Endian Swap/1(I) -> Logical3/2
  Delay/1 -> Gateway Out10/1, crc_accum/3
  Mux/1 -> byte/1
  CRC8 Lookup/1 -> crc_accum/1
  Gateway Out4/1 -> HT-SIG CRC/5  "CRC"
  Constant/1 -> Relational1/2
  Gateway Out3/1 -> HT-SIG CRC/4  "Good"
  Gateway Out2/1 -> HT-SIG CRC/3  "Byte"
  Gateway Out1/1 -> HT-SIG CRC/2  "Reset"
  Gateway Out10/1 -> HT-SIG CRC/1  "Vin"
  Relational1/1 -> Gateway Out3/1, CRC Good/1
  byte/1 -> Endian Swap/1(B), Gateway Out2/1
  Assert1/1 -> Logical3/1, Gateway Out4/1, Relational1/1
  crc_accum/1 -> Assert1/1
  Logical3/1 -> CRC8 Lookup/1
  data_byte/1 -> Mux/2, 2LSB2/1, 6MSB/1, 2LSB3/1
  Concat2/1 -> Mux/4
  Concat4/1 -> Mux/3
  byte_ind/1 -> Relational5/1, Relational2/2, Relational4/1, Relational3/1
  Relational5/1 -> Concat1/2
  Constant5/1 -> Relational5/2
  Constant2/1 -> Relational2/1
  Relational2/1 -> Concat1/1
  Concat1/1 -> Mux/1
  Constant3/1 -> Relational3/2
  Constant4/1 -> Relational4/2
  data_valid/1 -> Logical1/1
  Relational3/1 -> Logical1/2
  Relational4/1 -> Logical1/3
  Logical1/1 -> Delay/1, byte/3
  reset/1 -> byte/2, crc_accum/2, Gateway Out1/1

== system wlan_phy_rx_pmd/Detect & Decode/Decode/SIGNAL & HT-SIG Decode/HT-SIG Decode/HT Length ==
  note: HT-SIG.LENGTH can be 0, indicating
      | a null-data frame used for channel sounding
      | (IEEE 802.11-2012 20.3.9.4.3)
      |
      | However our MAC/PHY do not currently support
      | the beamforming modes that use NDP packets
      | for channel sounding. So we require a non-zero
      | HT-SIG.LENGTH for any packet that is passed
      | to the lower MAC code. This check in hardware
      | reduces the impact of bad SIGNAL fields with
      | bogus, small LENGTH fields. The downstream
      | logic assumes every packet will have at least
      | 6 bytes after the SIGNAL field.
  B4 : Inport
  B5 : Inport  Port=2
  Concat1 : xbsIndex_r4/Concat  num_inputs=2  dbl_ovrd=off  block_type=concat
  Concat2 : xbsIndex_r4/Concat  num_inputs=2  dbl_ovrd=off  block_type=concat
  Constant : xbsIndex_r4/Constant  const=0  gui_display_data_type=Fixed-point  arith_type=Unsigned  n_bits=10  bin_pt=0  preci_type=Single  exp_width=8  frac_width=24  explicit_period=off  period=1  equ=P=C  opselect=C  inp2=PCIN>>17  opr=+  inp1=P  carry=CIN  dbl_ovrd=off  block_type=constant
  Constant1 : xbsIndex_r4/Constant  const=6  gui_display_data_type=Fixed-point  arith_type=Unsigned  n_bits=3  bin_pt=0  preci_type=Single  exp_width=8  frac_width=24  explicit_period=off  period=1  equ=P=C  opselect=C  inp2=PCIN>>17  opr=+  inp1=P  carry=CIN  dbl_ovrd=off  block_type=constant
  Logical : xbsIndex_r4/Logical  logical_function=OR  inputs=2  en=off  latency=0  precision=Full  arith_type=Unsigned  n_bits=16  bin_pt=0  align_bp=on  dbl_ovrd=off  block_type=logical
  Register1 : xbsIndex_r4/Register  init=0  rst=off  en=off  dbl_ovrd=off  block_type=register
  Relational1 : xbsIndex_r4/Relational  mode=a<b  en=off  latency=0  dbl_ovrd=off  block_type=relational
  Relational2 : xbsIndex_r4/Relational  mode=a>b  en=off  latency=0  dbl_ovrd=off  block_type=relational
  length : Outport
  Unsupported : Outport  Port=2
  wires:
  B5/1 -> Concat1/1
  B4/1 -> Concat1/2
  Concat1/1 -> length/1, Relational2/1, Relational1/1
  [regRx_MAX_SIGNAL_LENGTH_KB] -> Register1/1
  Constant/1 -> Concat2/2
  Relational2/1 -> Logical/1
  Concat2/1 -> Relational2/2
  Constant1/1 -> Relational1/2
  Register1/1 -> Concat2/1
  Relational1/1 -> Logical/2
  Logical/1 -> Unsupported/1

== system wlan_phy_rx_pmd/Detect & Decode/Decode/SIGNAL & HT-SIG Decode/HT-SIG Decode/HT-SIG Flags ==
  note: HT-SIG Flags
      | (IEEE 802.11-2012 Figured 20-6)
      |
      | B3[7]: Channel bandwidth (0=20, 1=40)
      |
      | B6[0]: Smoothing
      | B6[1]: Not sounding
      | B6[2]: Reserved (always 1)
      | B6[3]: Aggregation
      | B6[5:4]: STBC
      | B6[6]: FEC (0=BCC, 1=LDPC)
      | B6[7]: Short GI
      |
      | B7[1:0]: Num extension spatial streams
  B3 : Inport
  B6 : Inport  Port=2
  B7 : Inport  Port=3
  Constant : xbsIndex_r4/Constant  const=0  gui_display_data_type=Fixed-point  arith_type=Unsigned  n_bits=1  bin_pt=0  preci_type=Single  exp_width=8  frac_width=24  explicit_period=off  period=1  equ=P=C  opselect=C  inp2=PCIN>>17  opr=+  inp1=P  carry=CIN  dbl_ovrd=off  block_type=constant
  Constant1 : xbsIndex_r4/Constant  const=0  gui_display_data_type=Fixed-point  arith_type=Unsigned  n_bits=1  bin_pt=0  preci_type=Single  exp_width=8  frac_width=24  explicit_period=off  period=1  equ=P=C  opselect=C  inp2=PCIN>>17  opr=+  inp1=P  carry=CIN  dbl_ovrd=off  block_type=constant
  Inverter : xbsIndex_r4/Inverter  en=off  latency=0  dbl_ovrd=off  block_type=inv
  Logical : xbsIndex_r4/Logical  logical_function=OR  inputs=6  en=off  latency=0  precision=Full  arith_type=Unsigned  n_bits=16  bin_pt=0  align_bp=on  dbl_ovrd=off  block_type=logical
  Relational : xbsIndex_r4/Relational  mode=a!=b  en=off  latency=0  dbl_ovrd=off  block_type=relational
  Relational1 : xbsIndex_r4/Relational  mode=a!=b  en=off  latency=0  dbl_ovrd=off  block_type=relational
  b[0] : xbsIndex_r4/Slice  nbits=1  boolean_output=on  mode=Lower Bit Location + Width  bit1=0  base1=MSB of Input  bit0=0  base0=LSB of Input  dbl_ovrd=off  block_type=slice
  b[1:0] : xbsIndex_r4/Slice  nbits=2  boolean_output=off  mode=Lower Bit Location + Width  bit1=0  base1=MSB of Input  bit0=0  base0=LSB of Input  dbl_ovrd=off  block_type=slice
  b[1] : xbsIndex_r4/Slice  nbits=1  boolean_output=on  mode=Lower Bit Location + Width  bit1=0  base1=MSB of Input  bit0=1  base0=LSB of Input  dbl_ovrd=off  block_type=slice
  b[2] : xbsIndex_r4/Slice  nbits=1  boolean_output=on  mode=Lower Bit Location + Width  bit1=0  base1=MSB of Input  bit0=1  base0=LSB of Input  dbl_ovrd=off  block_type=slice
  b[3] : xbsIndex_r4/Slice  nbits=1  boolean_output=on  mode=Lower Bit Location + Width  bit1=0  base1=MSB of Input  bit0=3  base0=LSB of Input  dbl_ovrd=off  block_type=slice
  b[5:4] : xbsIndex_r4/Slice  nbits=2  boolean_output=off  mode=Lower Bit Location + Width  bit1=0  base1=MSB of Input  bit0=4  base0=LSB of Input  dbl_ovrd=off  block_type=slice
  b[6] : xbsIndex_r4/Slice  nbits=1  boolean_output=on  mode=Lower Bit Location + Width  bit1=0  base1=MSB of Input  bit0=6  base0=LSB of Input  dbl_ovrd=off  block_type=slice
  b[7] : xbsIndex_r4/Slice  nbits=1  boolean_output=on  mode=Lower Bit Location + Width  bit1=0  base1=MSB of Input  bit0=7  base0=LSB of Input  dbl_ovrd=off  block_type=slice
  "b[7] " : xbsIndex_r4/Slice  nbits=1  boolean_output=on  mode=Lower Bit Location + Width  bit1=0  base1=MSB of Input  bit0=7  base0=LSB of Input  dbl_ovrd=off  block_type=slice
  Unsupported : Outport
  Valid : Outport  Port=2
  wires:
  B3/1 -> b[7]/1
  B6/1 -> b[0]/1, b[1]/1, b[2]/1, b[3]/1, b[5:4]/1, b[6]/1, "b[7] "/1
  b[7]/1 -> [CHAN_BW], Logical/1
  b[3]/1 -> [AGGREGATION]
  b[1]/1 -> [NOT_SOUNDING], Inverter/1
  b[0]/1 -> [SMOOTHING]
  b[5:4]/1 -> [STBC], Relational1/1
  b[6]/1 -> [FEC], Logical/3
  "b[7] "/1 -> [SHORT_GI], Logical/4
  B7/1 -> b[1:0]/1
  b[1:0]/1 -> [N_ESS], Relational/1
  Constant/1 -> Relational/2
  Constant1/1 -> Relational1/2
  Logical/1 -> Unsupported/1
  Relational1/1 -> Logical/5
  Relational/1 -> Logical/6
  Inverter/1 -> Logical/2
  b[2]/1 -> Valid/1
